simulink model "SpeedOptimisedVersionTannenweg"  (R2024a)
settings: Solver=ode15s  StartTime=0.0  StopTime=3600*2  FixedStep=0.1  RelTol=0.001
model workspace (MAT data in the model file):
  Ti = 5
  fa = 0.00071
  fd = 0.03
  ips = 36
  ma = 0.0013
  md = 0.04
  roomarea1 = 20
  s = 15
libraries: fl_lib x1434, nesl_utility x105, SimscapeFluids_lib x35
(part 2 of 22)

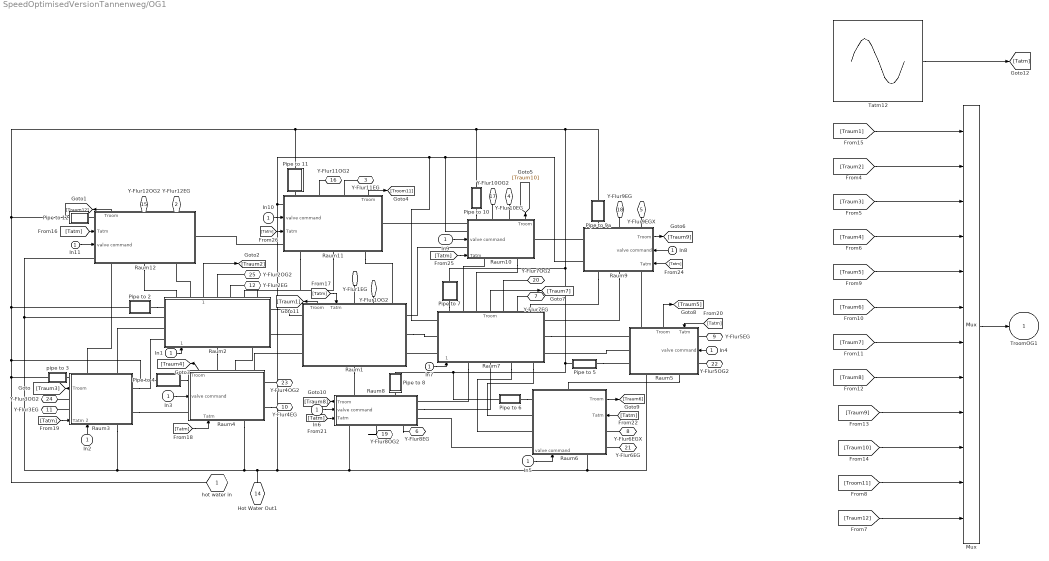
== system SpeedOptimisedVersionTannenweg/OG1 ==
  In1 : Inport
  In{2..11} (10 alike) : InportShadow
  Mux : Mux  Inputs=12
  Pipe to 10 : Subsystem
  Pipe to 11 : Subsystem
  Pipe to 12 : Subsystem
  Pipe to 2 : Subsystem
  Pipe to 4 : Subsystem
  Pipe to 5 : Subsystem
  Pipe to 6 : Subsystem
  Pipe to 7 : Subsystem
  Pipe to 8 : Subsystem
  Pipe to 9a : Subsystem
  Raum1 : Subsystem
      settings: PortSchema="{\"entries\":[{\"content\":{\"sides\":[{\"content\":{\"connectorIds\":[\"LConn1\",\"LConn2\",\"LConn3\"]},\"type\":\"ConnectorPlacement.EquallySpacedRectSide\",\"uuid\":\"258852ae-79b4-4e90-ae22-4154c858ab0a\"},{\"content\":{\"connectorIds\":[\"RConn1\",\"RConn2\",\"RConn3\"],\"side\":\"RIGHT\"},\"type\":\"ConnectorPlacement.EquallySpacedRectSide\",\"uuid\":\"36706832-f330-42ce-9a32-f145cdee4912\"},{\"content\":{\"connectorIds\":[\"LConn4\",\"LConn5\",\"LConn6\",\"In1\",\"Out1\"],\"side\":\"TOP\"},\"type\":\"ConnectorPlacement.EquallySpacedRectSide\",\"uuid\":\"5cf2347a-3520-447e-9561-2d1c8313f3cf\"},{\"content\":{\"connectorIds\":[\"RConn4\"],\"side\":\"BOTTOM\"},\"type\":\"ConnectorPlacement.EquallySpacedRectSide\",\"uuid\":\"b1588268-8d41-4423-8f6e-2c28bf9b13dc\"}]},\"type\":\"ConnectorPlacement.EquallySpacedConnector\",\"uuid\":\"6bed35e7-1d7a-421b-a1f8-39267ed9017d\"}],\"packageUris\":[\"http://schema.mathworks.com/mf0/Connector/R2020b\"],\"version\":\"1.0\"}"
  Raum10 : Subsystem
      settings: PortSchema="{\"entries\":[{\"content\":{\"sides\":[{\"content\":{\"connectorIds\":[\"LConn1\",\"LConn2\",\"In1\",\"LConn3\",\"In2\"]},\"type\":\"ConnectorPlacement.EquallySpacedRectSide\",\"uuid\":\"258852ae-79b4-4e90-ae22-4154c858ab0a\"},{\"content\":{\"connectorIds\":[\"RConn1\"],\"side\":\"RIGHT\"},\"type\":\"ConnectorPlacement.EquallySpacedRectSide\",\"uuid\":\"36706832-f330-42ce-9a32-f145cdee4912\"},{\"content\":{\"connectorIds\":[\"LConn4\",\"LConn5\",\"LConn6\",\"Out1\"],\"side\":\"TOP\"},\"type\":\"ConnectorPlacement.EquallySpacedRectSide\",\"uuid\":\"5cf2347a-3520-447e-9561-2d1c8313f3cf\"},{\"content\":{\"connectorIds\":[\"RConn2\",\"RConn3\"],\"side\":\"BOTTOM\"},\"type\":\"ConnectorPlacement.EquallySpacedRectSide\",\"uuid\":\"b1588268-8d41-4423-8f6e-2c28bf9b13dc\"}]},\"type\":\"ConnectorPlacement.EquallySpacedConnector\",\"uuid\":\"6bed35e7-1d7a-421b-a1f8-39267ed9017d\"}],\"packageUris\":[\"http://schema.mathworks.com/mf0/Connector/R2020b\"],\"version\":\"1.0\"}"
  Raum11 : Subsystem
      settings: PortSchema="{\"entries\":[{\"content\":{\"sides\":[{\"content\":{\"connectorIds\":[\"LConn1\",\"In1\",\"In2\",\"LConn2\"]},\"type\":\"ConnectorPlacement.EquallySpacedRectSide\",\"uuid\":\"258852ae-79b4-4e90-ae22-4154c858ab0a\"},{\"content\":{\"connectorIds\":[\"RConn1\"],\"side\":\"RIGHT\"},\"type\":\"ConnectorPlacement.EquallySpacedRectSide\",\"uuid\":\"36706832-f330-42ce-9a32-f145cdee4912\"},{\"content\":{\"connectorIds\":[\"LConn3\",\"LConn4\",\"LConn5\",\"Out1\"],\"side\":\"TOP\"},\"type\":\"ConnectorPlacement.EquallySpacedRectSide\",\"uuid\":\"5cf2347a-3520-447e-9561-2d1c8313f3cf\"},{\"content\":{\"connectorIds\":[\"RConn2\",\"RConn3\",\"RConn4\"],\"side\":\"BOTTOM\"},\"type\":\"ConnectorPlacement.EquallySpacedRectSide\",\"uuid\":\"b1588268-8d41-4423-8f6e-2c28bf9b13dc\"}]},\"type\":\"ConnectorPlacement.EquallySpacedConnector\",\"uuid\":\"6bed35e7-1d7a-421b-a1f8-39267ed9017d\"}],\"packageUris\":[\"http://schema.mathworks.com/mf0/Connector/R2020b\"],\"version\":\"1.0\"}"
  Raum12 : Subsystem
      settings: PortSchema="{\"entries\":[{\"content\":{\"sides\":[{\"content\":{\"connectorIds\":[\"LConn1\",\"In1\",\"In2\",\"LConn2\"]},\"type\":\"ConnectorPlacement.EquallySpacedRectSide\",\"uuid\":\"258852ae-79b4-4e90-ae22-4154c858ab0a\"},{\"content\":{\"connectorIds\":[\"RConn1\"],\"side\":\"RIGHT\"},\"type\":\"ConnectorPlacement.EquallySpacedRectSide\",\"uuid\":\"36706832-f330-42ce-9a32-f145cdee4912\"},{\"content\":{\"connectorIds\":[\"Out1\",\"LConn3\",\"LConn4\"],\"side\":\"TOP\"},\"type\":\"ConnectorPlacement.EquallySpacedRectSide\",\"uuid\":\"5cf2347a-3520-447e-9561-2d1c8313f3cf\"},{\"content\":{\"connectorIds\":[\"RConn2\",\"RConn3\",\"RConn4\"],\"side\":\"BOTTOM\"},\"type\":\"ConnectorPlacement.EquallySpacedRectSide\",\"uuid\":\"b1588268-8d41-4423-8f6e-2c28bf9b13dc\"}]},\"type\":\"ConnectorPlacement.EquallySpacedConnector\",\"uuid\":\"6bed35e7-1d7a-421b-a1f8-39267ed9017d\"}],\"packageUris\":[\"http://schema.mathworks.com/mf0/Connector/R2020b\"],\"version\":\"1.0\"}"
  Raum2 : Subsystem
      settings: PortSchema="{\"entries\":[{\"content\":{\"sides\":[{\"content\":{\"connectorIds\":[\"LConn1\",\"LConn2\",\"LConn3\",\"LConn4\"]},\"type\":\"ConnectorPlacement.EquallySpacedRectSide\",\"uuid\":\"258852ae-79b4-4e90-ae22-4154c858ab0a\"},{\"content\":{\"connectorIds\":[\"RConn1\",\"RConn2\"],\"side\":\"RIGHT\"},\"type\":\"ConnectorPlacement.EquallySpacedRectSide\",\"uuid\":\"36706832-f330-42ce-9a32-f145cdee4912\"},{\"content\":{\"connectorIds\":[\"LConn5\",\"LConn6\",\"Out1\",\"LConn7\",\"LConn8\",\"LConn9\",\"LConn10\"],\"side\":\"TOP\"},\"type\":\"ConnectorPlacement.EquallySpacedRectSide\",\"uuid\":\"5cf2347a-3520-447e-9561-2d1c8313f3cf\"},{\"content\":{\"connectorIds\":[\"In1\",\"RConn3\",\"RConn4\"],\"side\":\"BOTTOM\"},\"type\":\"ConnectorPlacement.EquallySpacedRectSide\",\"uuid\":\"b1588268-8d41-4423-8f6e-2c28bf9b13dc\"}]},\"type\":\"ConnectorPlacement.EquallySpacedConnector\",\"uuid\":\"6bed35e7-1d7a-421b-a1f8-39267ed9017d\"}],\"packageUris\":[\"http://schema.mathworks.com/mf0/Connector/R2020b\"],\"version\":\"1.0\"}"
  Raum3 : Subsystem
      settings: PortSchema="{\"entries\":[{\"content\":{\"sides\":[{\"content\":{\"connectorIds\":[\"LConn1\",\"Out1\",\"LConn2\",\"LConn3\",\"In1\"]},\"type\":\"ConnectorPlacement.EquallySpacedRectSide\",\"uuid\":\"258852ae-79b4-4e90-ae22-4154c858ab0a\"},{\"content\":{\"connectorIds\":[\"RConn1\",\"RConn2\"],\"side\":\"RIGHT\"},\"type\":\"ConnectorPlacement.EquallySpacedRectSide\",\"uuid\":\"36706832-f330-42ce-9a32-f145cdee4912\"},{\"content\":{\"connectorIds\":[\"LConn4\",\"LConn5\"],\"side\":\"TOP\"},\"type\":\"ConnectorPlacement.EquallySpacedRectSide\",\"uuid\":\"5cf2347a-3520-447e-9561-2d1c8313f3cf\"},{\"content\":{\"connectorIds\":[\"In2\",\"RConn3\"],\"side\":\"BOTTOM\"},\"type\":\"ConnectorPlacement.EquallySpacedRectSide\",\"uuid\":\"b1588268-8d41-4423-8f6e-2c28bf9b13dc\"}]},\"type\":\"ConnectorPlacement.EquallySpacedConnector\",\"uuid\":\"6bed35e7-1d7a-421b-a1f8-39267ed9017d\"}],\"packageUris\":[\"http://schema.mathworks.com/mf0/Connector/R2020b\"],\"version\":\"1.0\"}"
  Raum4 : Subsystem
      settings: PortSchema="{\"entries\":[{\"content\":{\"sides\":[{\"content\":{\"connectorIds\":[\"LConn1\",\"In1\",\"LConn2\"]},\"type\":\"ConnectorPlacement.EquallySpacedRectSide\",\"uuid\":\"258852ae-79b4-4e90-ae22-4154c858ab0a\"},{\"content\":{\"connectorIds\":[\"RConn1\",\"RConn2\"],\"side\":\"RIGHT\"},\"type\":\"ConnectorPlacement.EquallySpacedRectSide\",\"uuid\":\"36706832-f330-42ce-9a32-f145cdee4912\"},{\"content\":{\"connectorIds\":[\"Out1\",\"LConn3\",\"LConn4\",\"LConn5\"],\"side\":\"TOP\"},\"type\":\"ConnectorPlacement.EquallySpacedRectSide\",\"uuid\":\"5cf2347a-3520-447e-9561-2d1c8313f3cf\"},{\"content\":{\"connectorIds\":[\"In2\",\"RConn3\"],\"side\":\"BOTTOM\"},\"type\":\"ConnectorPlacement.EquallySpacedRectSide\",\"uuid\":\"b1588268-8d41-4423-8f6e-2c28bf9b13dc\"}]},\"type\":\"ConnectorPlacement.EquallySpacedConnector\",\"uuid\":\"6bed35e7-1d7a-421b-a1f8-39267ed9017d\"}],\"packageUris\":[\"http://schema.mathworks.com/mf0/Connector/R2020b\"],\"version\":\"1.0\"}"
  Raum5 : Subsystem
      settings: PortSchema="{\"entries\":[{\"content\":{\"sides\":[{\"content\":{\"connectorIds\":[\"LConn1\",\"LConn2\",\"LConn3\"]},\"type\":\"ConnectorPlacement.EquallySpacedRectSide\",\"uuid\":\"258852ae-79b4-4e90-ae22-4154c858ab0a\"},{\"content\":{\"connectorIds\":[\"RConn1\",\"In2\",\"RConn2\"],\"side\":\"RIGHT\"},\"type\":\"ConnectorPlacement.EquallySpacedRectSide\",\"uuid\":\"36706832-f330-42ce-9a32-f145cdee4912\"},{\"content\":{\"connectorIds\":[\"LConn4\",\"Out1\",\"In1\"],\"side\":\"TOP\"},\"type\":\"ConnectorPlacement.EquallySpacedRectSide\",\"uuid\":\"5cf2347a-3520-447e-9561-2d1c8313f3cf\"},{\"content\":{\"connectorIds\":[\"RConn3\",\"RConn4\"],\"side\":\"BOTTOM\"},\"type\":\"ConnectorPlacement.EquallySpacedRectSide\",\"uuid\":\"b1588268-8d41-4423-8f6e-2c28bf9b13dc\"}]},\"type\":\"ConnectorPlacement.EquallySpacedConnector\",\"uuid\":\"6bed35e7-1d7a-421b-a1f8-39267ed9017d\"}],\"packageUris\":[\"http://schema.mathworks.com/mf0/Connector/R2020b\"],\"version\":\"1.0\"}"
  Raum6 : Subsystem
      settings: PortSchema="{\"entries\":[{\"content\":{\"sides\":[{\"content\":{\"connectorIds\":[\"LConn1\",\"LConn2\",\"LConn3\",\"LConn4\"]},\"type\":\"ConnectorPlacement.EquallySpacedRectSide\",\"uuid\":\"258852ae-79b4-4e90-ae22-4154c858ab0a\"},{\"content\":{\"connectorIds\":[\"Out1\",\"In2\",\"RConn1\",\"RConn2\"],\"side\":\"RIGHT\"},\"type\":\"ConnectorPlacement.EquallySpacedRectSide\",\"uuid\":\"36706832-f330-42ce-9a32-f145cdee4912\"},{\"content\":{\"connectorIds\":[\"LConn5\"],\"side\":\"TOP\"},\"type\":\"ConnectorPlacement.EquallySpacedRectSide\",\"uuid\":\"5cf2347a-3520-447e-9561-2d1c8313f3cf\"},{\"content\":{\"connectorIds\":[\"In1\",\"RConn3\"],\"side\":\"BOTTOM\"},\"type\":\"ConnectorPlacement.EquallySpacedRectSide\",\"uuid\":\"b1588268-8d41-4423-8f6e-2c28bf9b13dc\"}]},\"type\":\"ConnectorPlacement.EquallySpacedConnector\",\"uuid\":\"6bed35e7-1d7a-421b-a1f8-39267ed9017d\"}],\"packageUris\":[\"http://schema.mathworks.com/mf0/Connector/R2020b\"],\"version\":\"1.0\"}"
  Raum7 : Subsystem
      settings: PortSchema="{\"entries\":[{\"content\":{\"sides\":[{\"content\":{\"connectorIds\":[\"LConn1\",\"LConn2\",\"LConn3\"]},\"type\":\"ConnectorPlacement.EquallySpacedRectSide\",\"uuid\":\"258852ae-79b4-4e90-ae22-4154c858ab0a\"},{\"content\":{\"connectorIds\":[\"RConn1\",\"RConn2\",\"RConn3\"],\"side\":\"RIGHT\"},\"type\":\"ConnectorPlacement.EquallySpacedRectSide\",\"uuid\":\"36706832-f330-42ce-9a32-f145cdee4912\"},{\"content\":{\"connectorIds\":[\"LConn4\",\"LConn5\",\"LConn6\",\"Out1\",\"LConn7\",\"LConn8\",\"LConn9\"],\"side\":\"TOP\"},\"type\":\"ConnectorPlacement.EquallySpacedRectSide\",\"uuid\":\"5cf2347a-3520-447e-9561-2d1c8313f3cf\"},{\"content\":{\"connectorIds\":[\"In1\",\"RConn4\",\"RConn5\",\"RConn6\",\"RConn7\"],\"side\":\"BOTTOM\"},\"type\":\"ConnectorPlacement.EquallySpacedRectSide\",\"uuid\":\"b1588268-8d41-4423-8f6e-2c28bf9b13dc\"}]},\"type\":\"ConnectorPlacement.EquallySpacedConnector\",\"uuid\":\"6bed35e7-1d7a-421b-a1f8-39267ed9017d\"}],\"packageUris\":[\"http://schema.mathworks.com/mf0/Connector/R2020b\"],\"version\":\"1.0\"}"
  Raum8 : Subsystem
      settings: PortSchema="{\"entries\":[{\"content\":{\"sides\":[{\"content\":{\"connectorIds\":[\"Out1\",\"In1\",\"In2\"]},\"type\":\"ConnectorPlacement.EquallySpacedRectSide\",\"uuid\":\"258852ae-79b4-4e90-ae22-4154c858ab0a\"},{\"content\":{\"connectorIds\":[\"RConn1\",\"RConn2\",\"RConn3\"],\"side\":\"RIGHT\"},\"type\":\"ConnectorPlacement.EquallySpacedRectSide\",\"uuid\":\"36706832-f330-42ce-9a32-f145cdee4912\"},{\"content\":{\"connectorIds\":[\"LConn1\",\"LConn2\"],\"side\":\"TOP\"},\"type\":\"ConnectorPlacement.EquallySpacedRectSide\",\"uuid\":\"5cf2347a-3520-447e-9561-2d1c8313f3cf\"},{\"content\":{\"connectorIds\":[\"RConn4\",\"RConn5\",\"RConn6\"],\"side\":\"BOTTOM\"},\"type\":\"ConnectorPlacement.EquallySpacedRectSide\",\"uuid\":\"b1588268-8d41-4423-8f6e-2c28bf9b13dc\"}]},\"type\":\"ConnectorPlacement.EquallySpacedConnector\",\"uuid\":\"6bed35e7-1d7a-421b-a1f8-39267ed9017d\"}],\"packageUris\":[\"http://schema.mathworks.com/mf0/Connector/R2020b\"],\"version\":\"1.0\"}"
  Raum9 : Subsystem
      settings: PortSchema="{\"entries\":[{\"content\":{\"sides\":[{\"content\":{\"connectorIds\":[\"LConn1\",\"LConn2\"]},\"type\":\"ConnectorPlacement.EquallySpacedRectSide\",\"uuid\":\"258852ae-79b4-4e90-ae22-4154c858ab0a\"},{\"content\":{\"connectorIds\":[\"Out1\",\"In1\",\"In2\"],\"side\":\"RIGHT\"},\"type\":\"ConnectorPlacement.EquallySpacedRectSide\",\"uuid\":\"36706832-f330-42ce-9a32-f145cdee4912\"},{\"content\":{\"connectorIds\":[\"LConn3\",\"LConn4\",\"LConn5\"],\"side\":\"TOP\"},\"type\":\"ConnectorPlacement.EquallySpacedRectSide\",\"uuid\":\"5cf2347a-3520-447e-9561-2d1c8313f3cf\"},{\"content\":{\"connectorIds\":[\"RConn1\",\"RConn2\",\"RConn3\"],\"side\":\"BOTTOM\"},\"type\":\"ConnectorPlacement.EquallySpacedRectSide\",\"uuid\":\"b1588268-8d41-4423-8f6e-2c28bf9b13dc\"}]},\"type\":\"ConnectorPlacement.EquallySpacedConnector\",\"uuid\":\"6bed35e7-1d7a-421b-a1f8-39267ed9017d\"}],\"packageUris\":[\"http://schema.mathworks.com/mf0/Connector/R2020b\"],\"version\":\"1.0\"}"
  Tatm12 : Sin  Amplitude=7  Frequency=2*pi/(24*3600)  Phase=pi  SampleTime=0
  pipe to 3 : Subsystem
  hot water in : PMIOPort  Side=Left
  Y-Flur12EG : PMIOPort  Port=2  Side=Left
  Y-Flur11EG : PMIOPort  Port=3  Side=Left
  Y-Flur10EG : PMIOPort  Port=4  Side=Left
  Y-Flur9EGX : PMIOPort  Port=5  Side=Left
  Y-Flur8EG : PMIOPort  Port=6  Side=Left
  Y-Flur7EG : PMIOPort  Port=7  Side=Left
  Y-Flur6EGX : PMIOPort  Port=8  Side=Left
  Y-Flur5EG : PMIOPort  Port=9  Side=Left
  Y-Flur4EG : PMIOPort  Port=10  Side=Left
  Y-Flur3EG : PMIOPort  Port=11  Side=Left
  Y-Flur2EG : PMIOPort  Port=12  Side=Left
  Y-Flur1EG : PMIOPort  Port=13  Side=Left
  Hot Water Out1 : PMIOPort  Port=14  Side=Right
  Y-Flur12OG2 : PMIOPort  Port=15  Side=Right
  Y-Flur11OG2 : PMIOPort  Port=16  Side=Right
  Y-Flur10OG2 : PMIOPort  Port=17  Side=Right
  Y-Flur9EG : PMIOPort  Port=18  Side=Right
  Y-Flur8OG2 : PMIOPort  Port=19  Side=Right
  Y-Flur7OG2 : PMIOPort  Port=20  Side=Right
  Y-Flur6EG : PMIOPort  Port=21  Side=Right
  Y-Flur5OG2 : PMIOPort  Port=22  Side=Right
  Y-Flur4OG2 : PMIOPort  Port=23  Side=Right
  Y-Flur3OG2 : PMIOPort  Port=24  Side=Right
  Y-Flur2OG2 : PMIOPort  Port=25  Side=Right
  Y-Flur1OG2 : PMIOPort  Port=26  Side=Right
  TroomOG1 : Outport
  wires:
  [Tatm] -> Raum12/1(Tatm)
  Raum12/1(Troom) -> [Traum12]
  Raum8/1(Troom) -> [Traum8]
  Raum5/1(Troom) -> [Traum5]
  Raum4/1(Troom) -> [Traum4]
  [Tatm] -> Raum8/2(Tatm)
  [Tatm] -> Raum6/2(Tatm)
  Raum1/1(Troom) -> [Traum1]
  Raum10/1(Troom) -> [Traum10]
  Raum3/1(Troom) -> [Traum3]
  [Tatm] -> Raum4/2(Tatm)
  [Tatm] -> Raum3/1(Tatm)
  [Tatm] -> Raum5/1(Tatm)
  [Tatm] -> Raum1/1(Tatm)
  [Tatm] -> Raum10/2(Tatm)
  Raum2/1(Troom) -> [Traum2]
  Raum11/1(Troom) -> [Troom11]
  Raum7/1(Troom) -> [Traum7]
  [Tatm] -> Raum9/2(Tatm)
  Raum6/1(Troom) -> [Traum6]
  [Tatm] -> Raum11/2(Tatm)
  Raum9/1(Troom) -> [Traum9]
  Tatm12/1 -> [Tatm]
  In1/1 -> Raum2/1(valve command)
  In2/1 -> Raum3/2(valve command)
  In3/1 -> Raum4/1(valve command)
  In6/1 -> Raum8/1(valve command)
  In5/1 -> Raum6/1(valve command)
  In4/1 -> Raum5/2(valve command)
  In7/1 -> Raum7/1(valve command)
  In8/1 -> Raum9/1(valve command)
  In9/1 -> Raum10/1(valve command)
  In10/1 -> Raum11/1(valve command)
  In11/1 -> Raum12/2(valve command)
  [Traum9] -> Mux/9
  [Traum7] -> Mux/7
  [Traum8] -> Mux/8
  [Traum12] -> Mux/12
  [Traum5] -> Mux/5
  [Traum6] -> Mux/6
  [Traum3] -> Mux/3
  [Traum4] -> Mux/4
  Mux/1 -> TroomOG1/1
  [Traum2] -> Mux/2
  [Traum1] -> Mux/1
  [Troom11] -> Mux/11
  [Traum10] -> Mux/10
  connections:
  Raum2/LConn7 -- Y-Flur2OG2/RConn1
  Raum3/LConn2 -- Y-Flur3OG2/RConn1
  Y-Flur4OG2/RConn1 -- Raum4/RConn1
  Raum1/LConn5 -- Y-Flur1OG2/RConn1
  Y-Flur8OG2/RConn1 -- Raum8/RConn5
  Y-Flur6EG/RConn1 -- Raum6/RConn2
  Raum7/LConn7 -- Y-Flur7OG2/RConn1
  Y-Flur5OG2/RConn1 -- Raum5/RConn2
  Raum9/LConn4 -- Y-Flur9EG/RConn1
  Raum10/LConn5 -- Y-Flur10OG2/RConn1
  Raum11/LConn4 -- Y-Flur11OG2/RConn1
  Raum12/LConn3 -- Y-Flur12OG2/RConn1
  Raum2/RConn1 -- Raum1/RConn1
  Raum7/LConn5 -- Raum10/RConn2
  Raum8/RConn2 -- Raum7/RConn5
  Raum11/RConn3 -- Raum2/LConn10
  Raum1/LConn2 -- Raum7/LConn2
  Raum1/RConn3 -- Raum4/LConn5
  Pipe to 5/RConn1 -- Raum5/LConn3
  Raum3/RConn2 -- Raum4/LConn2
  Raum9/RConn1 -- Raum7/LConn9
  Raum3/RConn1 -- Raum2/LConn4
  Raum11/RConn2 -- Raum2/LConn9
  Raum7/RConn2 -- Raum5/LConn1
  Raum10/RConn3 -- Raum7/LConn6
  Raum12/RConn3 -- Raum2/LConn5
  Pipe to 2/RConn1 -- Raum2/LConn1
  Raum10/LConn3 -- Raum1/LConn1
  Raum3/LConn4 -- Raum12/RConn2
  Raum7/RConn4 -- Raum8/RConn1
  Raum2/RConn3 -- Raum4/LConn3
  Raum5/LConn2 -- Raum7/RConn3
  Raum9/LConn1 -- Raum10/RConn1
  Raum1/RConn4 -- Raum8/LConn1
  pipe to 3/RConn1 -- Raum3/LConn1
  Pipe to 10/RConn1 -- Raum10/LConn4
  Raum7/LConn3 -- Raum1/LConn3
  Raum2/LConn6 -- Raum12/RConn4
  Raum10/LConn1 -- Raum11/RConn1
  Raum9/RConn2 -- Raum7/RConn1
  Pipe to 11/RConn1 -- Raum11/LConn3
  Pipe to 8/RConn1 -- Raum8/LConn2
  Raum7/RConn7 -- Raum6/LConn2
  Pipe to 4/RConn1 -- Raum4/LConn1
  Raum5/LConn4 -- Raum9/RConn3
  Raum9/LConn3 -- Pipe to 9a/RConn1
  Raum11/RConn4 -- Raum1/LConn4
  Raum4/LConn4 -- Raum2/RConn4
  Raum3/LConn5 -- Raum2/LConn3
  Raum11/LConn2 -- Raum12/RConn1
  Pipe to 7/RConn1 -- Raum7/LConn4
  Pipe to 12/RConn1 -- Raum12/LConn1
  Raum1/RConn2 -- Raum2/RConn2
  Pipe to 6/RConn1 -- Raum6/LConn1
  Raum7/RConn6 -- Raum6/LConn3
  Raum5/RConn4 -- Raum6/LConn5
  Raum11/LConn1 -- Raum9/LConn2 -- Raum10/LConn2 -- Raum7/LConn1 -- Raum4/RConn3 -- Raum3/RConn3 -- Raum2/LConn2 -- Raum12/LConn2 -- Hot Water Out1/RConn1 -- Raum5/RConn3 -- Raum6/RConn3 -- Raum8/RConn4
  Raum6/LConn4 -- Raum8/RConn3
  Raum1/LConn6 -- Y-Flur1EG/RConn1
  Raum3/LConn3 -- Y-Flur3EG/RConn1
  Raum4/RConn2 -- Y-Flur4EG/RConn1
  Y-Flur5EG/RConn1 -- Raum5/RConn1
  Y-Flur6EGX/RConn1 -- Raum6/RConn1
  Raum7/LConn8 -- Y-Flur7EG/RConn1
  Raum8/RConn6 -- Y-Flur8EG/RConn1
  Raum9/LConn5 -- Y-Flur9EGX/RConn1
  Raum10/LConn6 -- Y-Flur10EG/RConn1
  Raum11/LConn5 -- Y-Flur11EG/RConn1
  Raum12/LConn4 -- Y-Flur12EG/RConn1
  Raum2/LConn8 -- Y-Flur2EG/RConn1
  Pipe to 9a/LConn1 -- hot water in/RConn1 -- pipe to 3/LConn1 -- Pipe to 4/LConn1 -- Pipe to 2/LConn1 -- Pipe to 12/LConn1 -- Pipe to 11/LConn1 -- Pipe to 10/LConn1 -- Pipe to 6/LConn1 -- Pipe to 8/LConn1 -- Pipe to 5/LConn1 -- Pipe to 7/LConn1

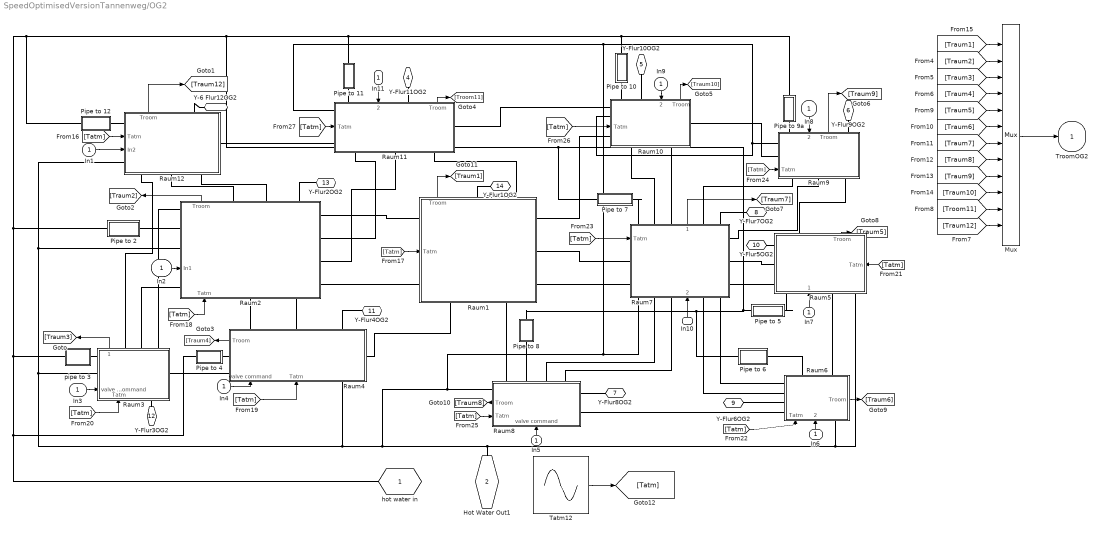
== system SpeedOptimisedVersionTannenweg/OG2 ==
  In1 : Inport
  In{2..11} (10 alike) : InportShadow
  Mux : Mux  Inputs=12
  Pipe to 10 : Subsystem
  Pipe to 11 : Subsystem
  Pipe to 12 : Subsystem
  Pipe to 2 : Subsystem
  Pipe to 4 : Subsystem
  Pipe to 5 : Subsystem
  Pipe to 6 : Subsystem
  Pipe to 7 : Subsystem
  Pipe to 8 : Subsystem
  Pipe to 9a : Subsystem
  Raum1 : Subsystem
      settings: PortSchema="{\"entries\":[{\"content\":{\"sides\":[{\"content\":{\"connectorIds\":[\"LConn1\",\"LConn2\",\"LConn3\"]},\"type\":\"ConnectorPlacement.EquallySpacedRectSide\",\"uuid\":\"258852ae-79b4-4e90-ae22-4154c858ab0a\"},{\"content\":{\"connectorIds\":[\"RConn1\",\"In1\",\"RConn2\"],\"side\":\"RIGHT\"},\"type\":\"ConnectorPlacement.EquallySpacedRectSide\",\"uuid\":\"36706832-f330-42ce-9a32-f145cdee4912\"},{\"content\":{\"connectorIds\":[\"LConn4\",\"LConn5\",\"Out1\"],\"side\":\"TOP\"},\"type\":\"ConnectorPlacement.EquallySpacedRectSide\",\"uuid\":\"5cf2347a-3520-447e-9561-2d1c8313f3cf\"},{\"content\":{\"connectorIds\":[\"RConn3\",\"RConn4\"],\"side\":\"BOTTOM\"},\"type\":\"ConnectorPlacement.EquallySpacedRectSide\",\"uuid\":\"b1588268-8d41-4423-8f6e-2c28bf9b13dc\"}]},\"type\":\"ConnectorPlacement.EquallySpacedConnector\",\"uuid\":\"6bed35e7-1d7a-421b-a1f8-39267ed9017d\"}],\"packageUris\":[\"http://schema.mathworks.com/mf0/Connector/R2020b\"],\"version\":\"1.0\"}"
  Raum10 : Subsystem
      settings: PortSchema="{\"entries\":[{\"content\":{\"sides\":[{\"content\":{\"connectorIds\":[\"LConn1\",\"LConn2\",\"In1\",\"LConn3\"]},\"type\":\"ConnectorPlacement.EquallySpacedRectSide\",\"uuid\":\"258852ae-79b4-4e90-ae22-4154c858ab0a\"},{\"content\":{\"connectorIds\":[\"RConn1\"],\"side\":\"RIGHT\"},\"type\":\"ConnectorPlacement.EquallySpacedRectSide\",\"uuid\":\"36706832-f330-42ce-9a32-f145cdee4912\"},{\"content\":{\"connectorIds\":[\"LConn4\",\"LConn5\",\"In2\",\"Out1\"],\"side\":\"TOP\"},\"type\":\"ConnectorPlacement.EquallySpacedRectSide\",\"uuid\":\"5cf2347a-3520-447e-9561-2d1c8313f3cf\"},{\"content\":{\"connectorIds\":[\"RConn2\",\"RConn3\"],\"side\":\"BOTTOM\"},\"type\":\"ConnectorPlacement.EquallySpacedRectSide\",\"uuid\":\"b1588268-8d41-4423-8f6e-2c28bf9b13dc\"}]},\"type\":\"ConnectorPlacement.EquallySpacedConnector\",\"uuid\":\"6bed35e7-1d7a-421b-a1f8-39267ed9017d\"}],\"packageUris\":[\"http://schema.mathworks.com/mf0/Connector/R2020b\"],\"version\":\"1.0\"}"
  Raum11 : Subsystem
      settings: PortSchema="{\"entries\":[{\"content\":{\"sides\":[{\"content\":{\"connectorIds\":[\"LConn1\",\"In1\",\"LConn2\"]},\"type\":\"ConnectorPlacement.EquallySpacedRectSide\",\"uuid\":\"258852ae-79b4-4e90-ae22-4154c858ab0a\"},{\"content\":{\"connectorIds\":[\"RConn1\"],\"side\":\"RIGHT\"},\"type\":\"ConnectorPlacement.EquallySpacedRectSide\",\"uuid\":\"36706832-f330-42ce-9a32-f145cdee4912\"},{\"content\":{\"connectorIds\":[\"LConn3\",\"In2\",\"LConn4\",\"Out1\"],\"side\":\"TOP\"},\"type\":\"ConnectorPlacement.EquallySpacedRectSide\",\"uuid\":\"5cf2347a-3520-447e-9561-2d1c8313f3cf\"},{\"content\":{\"connectorIds\":[\"RConn2\",\"RConn3\",\"RConn4\"],\"side\":\"BOTTOM\"},\"type\":\"ConnectorPlacement.EquallySpacedRectSide\",\"uuid\":\"b1588268-8d41-4423-8f6e-2c28bf9b13dc\"}]},\"type\":\"ConnectorPlacement.EquallySpacedConnector\",\"uuid\":\"6bed35e7-1d7a-421b-a1f8-39267ed9017d\"}],\"packageUris\":[\"http://schema.mathworks.com/mf0/Connector/R2020b\"],\"version\":\"1.0\"}"
  Raum12 : Subsystem
      settings: PortSchema="{\"entries\":[{\"content\":{\"sides\":[{\"content\":{\"connectorIds\":[\"LConn1\",\"In1\",\"In2\",\"LConn2\"]},\"type\":\"ConnectorPlacement.EquallySpacedRectSide\",\"uuid\":\"258852ae-79b4-4e90-ae22-4154c858ab0a\"},{\"content\":{\"connectorIds\":[\"RConn1\"],\"side\":\"RIGHT\"},\"type\":\"ConnectorPlacement.EquallySpacedRectSide\",\"uuid\":\"36706832-f330-42ce-9a32-f145cdee4912\"},{\"content\":{\"connectorIds\":[\"Out1\",\"LConn3\"],\"side\":\"TOP\"},\"type\":\"ConnectorPlacement.EquallySpacedRectSide\",\"uuid\":\"5cf2347a-3520-447e-9561-2d1c8313f3cf\"},{\"content\":{\"connectorIds\":[\"RConn2\",\"RConn3\",\"RConn4\"],\"side\":\"BOTTOM\"},\"type\":\"ConnectorPlacement.EquallySpacedRectSide\",\"uuid\":\"b1588268-8d41-4423-8f6e-2c28bf9b13dc\"}]},\"type\":\"ConnectorPlacement.EquallySpacedConnector\",\"uuid\":\"6bed35e7-1d7a-421b-a1f8-39267ed9017d\"}],\"packageUris\":[\"http://schema.mathworks.com/mf0/Connector/R2020b\"],\"version\":\"1.0\"}"